simulink model "r_128w_1k_1a_16x_r430"  (Simulink 7.2)
settings: Solver=FixedStepDiscrete  SolverMode=SingleTasking  StartTime=0.0  StopTime=5000  RelTol=1e-3
undefined symbols (not defined in the file): Down, pfb_coeff_gen_calc, Up
libraries: xbsIndex_r4 x8495, casper_library_misc x233, casper_library_delays x150, xps_library x70, simulink x28, dspsigops x12, casper_library_flow_control x8, casper_library_multipliers x8, xbsReplacements_r4 x4, casper_library_ffts_twiddle x2
(part 77 of 112)

== system r_128w_1k_1a_16x_r430/fft_wideband_real1/fft_direct/butterfly1_0/twiddle_general_4mult/coeff_gen ==
  rst : Inport
  Counter : xbsIndex_r4/Counter  cnt_type=Free Running  cnt_to=Inf  operation=Up  start_count=0  cnt_by_val=1  arith_type=Unsigned  n_bits=9  bin_pt=0  load_pin=off  rst=on  en=off  explicit_period=on  period=1  dbl_ovrd=off  use_behavioral_HDL=off  implementation=Fabric  block_type=counter
      settings: block_version=11.4
  ROM : xbsIndex_r4/ROM  depth=512  initVector="[1                     0.9999847412109375                     0.9999237060546875                     0.9998321533203125                       0.99969482421875                     0.9995269775390625                       0.99932861328125                       0.99908447265625                     0.9987945556640625                       0.99847412109375                     0.9981231689453125                     0.9977264404296875                      0.997283935546875                         0.996826171875                      0.996307373046875                     0.9957733154296875                       0.99517822265625                       0.99456787109375                     0.9939117431640625                     0.9932098388671875                     0.9924774169921875                     0.9917144775390625                       0.99090576171875                       0.99005126953125                     0.9891815185546875                      0.988250732421875                           0.9873046875                     0.9863128662109375                     0.9852752685546875                     0.9842071533203125                     0.9831085205078125                      0.981964111328125                     0.9807891845703125                     0.9795684814453125                     0.9783172607421875                     0.9770355224609375                        0.9757080078125                      0.974334716796875                     0.9729461669921875                       0.97149658203125                       0.97003173828125                     0.9685211181640625                       0.96697998046875                       0.96539306640625                      0.963775634765625                      0.962127685546875                     0.9604339599609375                      0.958709716796875                      0.956939697265625                       0.95513916015625                       0.95330810546875                     0.9514312744140625                       0.94952392578125                     0.9475860595703125                     0.9456024169921875                     0.9435882568359375                     0.9415435791015625                            0.939453125                     0.9373321533203125                        0.9351806640625                     0.9329986572265625                     0.9307708740234375                     0.9285125732421875                       0.92620849609375                     0.9238739013671875                        0.9215087890625                     0.9191131591796875                     0.9166717529296875                      0.914215087890625                      0.911712646484375                     0.9091644287109375                     0.9066009521484375                       0.90399169921875                     0.9013519287109375                         0.898681640625                      0.895965576171875                      0.893218994140625                       0.89044189453125                       0.88763427734375                      0.884796142578125                      0.881927490234375                     0.8790130615234375                      0.876068115234375                     0.8730926513671875                      0.870086669921875                     0.8670501708984375                     0.8639678955078125                      0.860870361328125                       0.85772705078125                       0.85455322265625                      0.851348876953125                      0.848114013671875                        0.8448486328125                         0.841552734375                      0.838226318359375                      0.834869384765625                     0.8314666748046875                     0.8280487060546875                        0.8245849609375                       0.82110595703125                     0.8175811767578125                     0.8140411376953125                      0.810455322265625 ...(+15932 chars)]"  distributed_mem=Block RAM  rst=off  init_reg=0  en=off  latency=2  arith_type="Signed  (2's comp)"  n_bits=18  bin_pt=16  dbl_ovrd=off  optimize=Area  use_rpm=on  block_type=sprom
      settings: block_version=11.4
  ROM1 : xbsIndex_r4/ROM  depth=512  initVector="[0                     -0.006134033203125                      -0.01226806640625                     -0.018402099609375                       -0.0245361328125                     -0.030670166015625                      -0.03680419921875                     -0.042938232421875                        -0.049072265625                    -0.0551910400390625                    -0.0613250732421875                      -0.06744384765625                    -0.0735626220703125                     -0.079681396484375                    -0.0858001708984375                    -0.0919036865234375                       -0.0980224609375                       -0.1041259765625                       -0.1102294921875                    -0.1163177490234375                     -0.122406005859375                    -0.1284942626953125                      -0.13458251953125                     -0.140655517578125                        -0.146728515625                     -0.152801513671875                    -0.1588592529296875                       -0.1649169921875                      -0.17095947265625                        -0.177001953125                      -0.18304443359375                    -0.1890716552734375                    -0.1950836181640625                      -0.20111083984375                    -0.2071075439453125                     -0.213104248046875                    -0.2191009521484375                    -0.2250823974609375                    -0.2310638427734375                     -0.237030029296875                      -0.24298095703125                     -0.248931884765625                    -0.2548675537109375                    -0.2607879638671875                    -0.2667083740234375                    -0.2726287841796875                    -0.2785186767578125                    -0.2844085693359375                        -0.290283203125                    -0.2961578369140625                        -0.302001953125                    -0.3078460693359375                    -0.3136749267578125                    -0.3195037841796875                       -0.3253173828125                    -0.3311004638671875                     -0.336883544921875                    -0.3426666259765625                     -0.348419189453125                     -0.354156494140625                     -0.359893798828125                    -0.3656158447265625                    -0.3713226318359375                      -0.37701416015625                       -0.3826904296875                    -0.3883514404296875                    -0.3939971923828125                     -0.399627685546875                     -0.405242919921875                    -0.4108428955078125                    -0.4164276123046875                       -0.4219970703125                      -0.42755126953125                    -0.4330902099609375                    -0.4386138916015625                     -0.444122314453125                     -0.449615478515625                           -0.455078125                     -0.460540771484375                     -0.465972900390625                    -0.4713897705078125                        -0.476806640625                        -0.482177734375                        -0.487548828125                    -0.4929046630859375                      -0.49822998046875                       -0.5035400390625                    -0.5088348388671875                      -0.51409912109375                    -0.5193634033203125                      -0.52459716796875                    -0.5298004150390625                     -0.535003662109375                    -0.5401763916015625                     -0.545318603515625                    -0.5504608154296875                     -0.555572509765625                       -0.5606689453125                      -0.56573486328125                    -0.5707855224609375                       -0.5758056640625                        -0.580810546875                    -0.5858001708984375 ...(+15932 chars)]"  distributed_mem=Block RAM  rst=off  init_reg=0  en=off  latency=2  arith_type="Signed  (2's comp)"  n_bits=18  bin_pt=16  dbl_ovrd=off  optimize=Area  use_rpm=on  block_type=sprom
      settings: block_version=11.4
  Slice : xbsIndex_r4/Slice  nbits=9  boolean_output=off  mode=Upper Bit Location + Width  bit1=0  base1=MSB of Input  bit0=0  base0=LSB of Input  dbl_ovrd=off  block_type=slice
  ri_to_c : casper_library_misc/ri_to_c  SystemSampleTime=-1
  w : Outport
  wires:
  ri_to_c/1 -> w/1
  rst/1 -> Counter/1
  Counter/1 -> Slice/1
  Slice/1 -> ROM/1, ROM1/1
  ROM/1 -> ri_to_c/1
  ROM1/1 -> ri_to_c/2

== system r_128w_1k_1a_16x_r430/fft_wideband_real1/fft_direct/butterfly1_1/convert_of0/convert ==
  note: (ones_bit & 1) -> round-to-even
      | (ones_bit ^ 1) -> round-to-odd
      | (sign_bit & 1)-> round-to-zero
      | (sign_bit ^ 1) -> round-to-inf
      | (X ~| 1) -> round half down
      | (X | 1) -> round half up
  in : Inport  OutDataType=sfix(16)  OutScaling=2^0
  Concat : xbsIndex_r4/Concat  num_inputs=2  dbl_ovrd=off  block_type=concat
      settings: block_version=10.1.3
  Constant : xbsIndex_r4/Constant  arith_type=Unsigned  const=1  n_bits=1  bin_pt=0  explicit_period=off  period=1  equ=P=C  opselect=C  inp2=PCIN>>17  opr=+  inp1=P  carry=CIN  dbl_ovrd=off  block_type=constant
  Reinterpret : xbsIndex_r4/Reinterpret  force_arith_type=on  arith_type=Unsigned  force_bin_pt=on  bin_pt=0  block_type=reinterpret
      settings: infoedit="Changes signal type without altering the binary representation.   You can changed the signal between signed and unsigned, and relocate the binary point.<P><P>Hardware notes: In hardware this block costs nothing.<P><P>Example:  Suppose the input is 6 bits wide, signed, with 2 fractional bits, and the output is forced to unsigned with 0 fractional bits.  Then an input of -2.0 (1110.00 in binary 2's complement) becomes an output of 56 (111000 in binary)."  block_version=10.1.3
  adder : xbsIndex_r4/AddSub  mode=Addition  use_carryin=off  use_carryout=off  en=off  latency=2  precision=User Defined  arith_type="Signed  (2's comp)"  n_bits=18  bin_pt=17  quantization=Truncate  overflow=Wrap  dbl_ovrd=off  use_behavioral_HDL=on  hw_selection=Fabric  pipelined=on  use_rpm=on  block_type=addsub
      settings: xl_area=[0, 0, 0, 0, 0, 0, 0]  block_version=10.1.3
  almost_half : xbsIndex_r4/Constant  arith_type=Unsigned  const=7  n_bits=21  bin_pt=0  explicit_period=off  period=1  equ=P=C  opselect=C  inp2=PCIN>>17  opr=+  inp1=P  carry=CIN  dbl_ovrd=off  block_type=constant
  bit : xbsIndex_r4/Slice  nbits=1  boolean_output=off  mode=Lower Bit Location + Width  bit1=0  base1=MSB of Input  bit0=3  base0=LSB of Input  dbl_ovrd=off  block_type=slice
      settings: infoedit="Extracts a given range of bits from each input sample and presents it at the output.  The output type is ordinarily unsigned with binary point at zero, but can be Boolean when the slice is one bit wide.<P><P>Hardware notes: In hardware this block costs nothing."  block_version=10.1.3
  force1 : xbsIndex_r4/Reinterpret  force_arith_type=on  arith_type="Signed  (2's comp)"  force_bin_pt=on  bin_pt=21  block_type=reinterpret
      settings: infoedit="Changes signal type without altering the binary representation.   You can changed the signal between signed and unsigned, and relocate the binary point.<P><P>Hardware notes: In hardware this block costs nothing.<P><P>Example:  Suppose the input is 6 bits wide, signed, with 2 fractional bits, and the output is forced to unsigned with 0 fractional bits.  Then an input of -2.0 (1110.00 in binary 2's complement) becomes an output of 56 (111000 in binary)."  block_version=10.1.3
  force2 : xbsIndex_r4/Reinterpret  force_arith_type=off  arith_type="Signed  (2's comp)"  force_bin_pt=on  bin_pt=21  block_type=reinterpret
      settings: infoedit="Changes signal type without altering the binary representation.   You can changed the signal between signed and unsigned, and relocate the binary point.<P><P>Hardware notes: In hardware this block costs nothing.<P><P>Example:  Suppose the input is 6 bits wide, signed, with 2 fractional bits, and the output is forced to unsigned with 0 fractional bits.  Then an input of -2.0 (1110.00 in binary 2's complement) becomes an output of 56 (111000 in binary)."  block_version=10.1.3
  tweak_op : xbsIndex_r4/Logical  logical_function=AND  inputs=2  en=off  latency=0  precision=Full  arith_type=Unsigned  n_bits=8  bin_pt=2  align_bp=on  dbl_ovrd=off  block_type=logical
      settings: xl_area=[0, 0, 0, 0, 0, 0, 0]
  out : Outport  OutDataType=sfix(16)  OutScaling=2^0
  wires:
  tweak_op/1 -> Concat/2
  Constant/1 -> tweak_op/2
  bit/1 -> tweak_op/1
  almost_half/1 -> force2/1
  in/1 -> Reinterpret/1, bit/1
  Concat/1 -> force1/1
  Reinterpret/1 -> Concat/1
  force1/1 -> adder/1
  force2/1 -> adder/2
  adder/1 -> out/1
